simulink model "CCMSysServ"  (R2022b) (continued)
== system CCMSysServ == (continued)
  CarCfg_CarCfg250/1 -> CCMSysServ_Runnable_sys/247(CarCfg_CarCfg250_read)
  CarCfg_CarCfg253/1 -> CCMSysServ_Runnable_sys/248(CarCfg_CarCfg253_read)
  CarCfg_CarCfg254/1 -> CCMSysServ_Runnable_sys/249(CarCfg_CarCfg254_read)
  CarCfg_CarCfg255/1 -> CCMSysServ_Runnable_sys/250(CarCfg_CarCfg255_read)
  CarCfg_CarCfg256/1 -> CCMSysServ_Runnable_sys/251(CarCfg_CarCfg256_read)
  CarCfg_CarCfg257/1 -> CCMSysServ_Runnable_sys/252(CarCfg_CarCfg257_read)
  CarCfg_CarCfg258/1 -> CCMSysServ_Runnable_sys/253(CarCfg_CarCfg258_read)
  CarCfg_CarCfg259/1 -> CCMSysServ_Runnable_sys/254(CarCfg_CarCfg259_read)
  CarCfg_CarCfg262/1 -> CCMSysServ_Runnable_sys/255(CarCfg_CarCfg262_read)
  CarCfg_CarCfg263/1 -> CCMSysServ_Runnable_sys/256(CarCfg_CarCfg263_read)
  CarCfg_CarCfg267/1 -> CCMSysServ_Runnable_sys/257(CarCfg_CarCfg267_read)
  CarCfg_CarCfg268/1 -> CCMSysServ_Runnable_sys/258(CarCfg_CarCfg268_read)
  CarCfg_CarCfg270/1 -> CCMSysServ_Runnable_sys/259(CarCfg_CarCfg270_read)
  CarCfg_CarCfg271/1 -> CCMSysServ_Runnable_sys/260(CarCfg_CarCfg271_read)
  CarCfg_CarCfg273/1 -> CCMSysServ_Runnable_sys/261(CarCfg_CarCfg273_read)
  CarCfg_CarCfg274/1 -> CCMSysServ_Runnable_sys/262(CarCfg_CarCfg274_read)
  CarCfg_CarCfg275/1 -> CCMSysServ_Runnable_sys/263(CarCfg_CarCfg275_read)
  CarCfg_CarCfg28/1 -> CCMSysServ_Runnable_sys/264(CarCfg_CarCfg28_read)
  CarCfg_CarCfg289/1 -> CCMSysServ_Runnable_sys/265(CarCfg_CarCfg289_read)
  CarCfg_CarCfg290/1 -> CCMSysServ_Runnable_sys/266(CarCfg_CarCfg290_read)
  CarCfg_CarCfg3/1 -> CCMSysServ_Runnable_sys/267(CarCfg_CarCfg3_read)
  CarCfg_CarCfg30/1 -> CCMSysServ_Runnable_sys/268(CarCfg_CarCfg30_read)
  CarCfg_CarCfg301/1 -> CCMSysServ_Runnable_sys/269(CarCfg_CarCfg301_read)
  CarCfg_CarCfg302/1 -> CCMSysServ_Runnable_sys/270(CarCfg_CarCfg302_read)
  CarCfg_CarCfg303/1 -> CCMSysServ_Runnable_sys/271(CarCfg_CarCfg303_read)
  CarCfg_CarCfg304/1 -> CCMSysServ_Runnable_sys/272(CarCfg_CarCfg304_read)
  CarCfg_CarCfg305/1 -> CCMSysServ_Runnable_sys/273(CarCfg_CarCfg305_read)
  CarCfg_CarCfg307/1 -> CCMSysServ_Runnable_sys/274(CarCfg_CarCfg307_read)
  CarCfg_CarCfg308/1 -> CCMSysServ_Runnable_sys/275(CarCfg_CarCfg308_read)
  CarCfg_CarCfg309/1 -> CCMSysServ_Runnable_sys/276(CarCfg_CarCfg309_read)
  CarCfg_CarCfg310/1 -> CCMSysServ_Runnable_sys/277(CarCfg_CarCfg310_read)
  CarCfg_CarCfg311/1 -> CCMSysServ_Runnable_sys/278(CarCfg_CarCfg311_read)
  CarCfg_CarCfg312/1 -> CCMSysServ_Runnable_sys/279(CarCfg_CarCfg312_read)
  CarCfg_CarCfg313/1 -> CCMSysServ_Runnable_sys/280(CarCfg_CarCfg313_read)
  CarCfg_CarCfg314/1 -> CCMSysServ_Runnable_sys/281(CarCfg_CarCfg314_read)
  CarCfg_CarCfg315/1 -> CCMSysServ_Runnable_sys/282(CarCfg_CarCfg315_read)
  CarCfg_CarCfg316/1 -> CCMSysServ_Runnable_sys/283(CarCfg_CarCfg316_read)
  CarCfg_CarCfg317/1 -> CCMSysServ_Runnable_sys/284(CarCfg_CarCfg317_read)
  CarCfg_CarCfg318/1 -> CCMSysServ_Runnable_sys/285(CarCfg_CarCfg318_read)
  CarCfg_CarCfg319/1 -> CCMSysServ_Runnable_sys/286(CarCfg_CarCfg319_read)
  CarCfg_CarCfg32/1 -> CCMSysServ_Runnable_sys/287(CarCfg_CarCfg32_read)
  CarCfg_CarCfg321/1 -> CCMSysServ_Runnable_sys/288(CarCfg_CarCfg321_read)
  CarCfg_CarCfg322/1 -> CCMSysServ_Runnable_sys/289(CarCfg_CarCfg322_read)
  CarCfg_CarCfg323/1 -> CCMSysServ_Runnable_sys/290(CarCfg_CarCfg323_read)
  CarCfg_CarCfg324/1 -> CCMSysServ_Runnable_sys/291(CarCfg_CarCfg324_read)
  CarCfg_CarCfg325/1 -> CCMSysServ_Runnable_sys/292(CarCfg_CarCfg325_read)
  CarCfg_CarCfg33/1 -> CCMSysServ_Runnable_sys/293(CarCfg_CarCfg33_read)
  CarCfg_CarCfg335/1 -> CCMSysServ_Runnable_sys/294(CarCfg_CarCfg335_read)
  CarCfg_CarCfg336/1 -> CCMSysServ_Runnable_sys/295(CarCfg_CarCfg336_read)
  CarCfg_CarCfg337/1 -> CCMSysServ_Runnable_sys/296(CarCfg_CarCfg337_read)
  CarCfg_CarCfg338/1 -> CCMSysServ_Runnable_sys/297(CarCfg_CarCfg338_read)
  CarCfg_CarCfg339/1 -> CCMSysServ_Runnable_sys/298(CarCfg_CarCfg339_read)
  CarCfg_CarCfg34/1 -> CCMSysServ_Runnable_sys/299(CarCfg_CarCfg34_read)
  CarCfg_CarCfg340/1 -> CCMSysServ_Runnable_sys/300(CarCfg_CarCfg340_read)
  CarCfg_CarCfg341/1 -> CCMSysServ_Runnable_sys/301(CarCfg_CarCfg341_read)
  CarCfg_CarCfg342/1 -> CCMSysServ_Runnable_sys/302(CarCfg_CarCfg342_read)
  CarCfg_CarCfg343/1 -> CCMSysServ_Runnable_sys/303(CarCfg_CarCfg343_read)
  CarCfg_CarCfg344/1 -> CCMSysServ_Runnable_sys/304(CarCfg_CarCfg344_read)
  CarCfg_CarCfg345/1 -> CCMSysServ_Runnable_sys/305(CarCfg_CarCfg345_read)
  CarCfg_CarCfg346/1 -> CCMSysServ_Runnable_sys/306(CarCfg_CarCfg346_read)
  CarCfg_CarCfg347/1 -> CCMSysServ_Runnable_sys/307(CarCfg_CarCfg347_read)
  CarCfg_CarCfg348/1 -> CCMSysServ_Runnable_sys/308(CarCfg_CarCfg348_read)
  CarCfg_CarCfg349/1 -> CCMSysServ_Runnable_sys/309(CarCfg_CarCfg349_read)
  CarCfg_CarCfg35/1 -> CCMSysServ_Runnable_sys/310(CarCfg_CarCfg35_read)
  CarCfg_CarCfg350/1 -> CCMSysServ_Runnable_sys/311(CarCfg_CarCfg350_read)
  CarCfg_CarCfg351/1 -> CCMSysServ_Runnable_sys/312(CarCfg_CarCfg351_read)
  CarCfg_CarCfg352/1 -> CCMSysServ_Runnable_sys/313(CarCfg_CarCfg352_read)
  CarCfg_CarCfg353/1 -> CCMSysServ_Runnable_sys/314(CarCfg_CarCfg353_read)
  CarCfg_CarCfg354/1 -> CCMSysServ_Runnable_sys/315(CarCfg_CarCfg354_read)
  CarCfg_CarCfg355/1 -> CCMSysServ_Runnable_sys/316(CarCfg_CarCfg355_read)
  CarCfg_CarCfg356/1 -> CCMSysServ_Runnable_sys/317(CarCfg_CarCfg356_read)
  CarCfg_CarCfg357/1 -> CCMSysServ_Runnable_sys/318(CarCfg_CarCfg357_read)
  CarCfg_CarCfg358/1 -> CCMSysServ_Runnable_sys/319(CarCfg_CarCfg358_read)
  CarCfg_CarCfg359/1 -> CCMSysServ_Runnable_sys/320(CarCfg_CarCfg359_read)
  CarCfg_CarCfg36/1 -> CCMSysServ_Runnable_sys/321(CarCfg_CarCfg36_read)
  CarCfg_CarCfg360/1 -> CCMSysServ_Runnable_sys/322(CarCfg_CarCfg360_read)
  CarCfg_CarCfg361/1 -> CCMSysServ_Runnable_sys/323(CarCfg_CarCfg361_read)
  CarCfg_CarCfg362/1 -> CCMSysServ_Runnable_sys/324(CarCfg_CarCfg362_read)
  CarCfg_CarCfg363/1 -> CCMSysServ_Runnable_sys/325(CarCfg_CarCfg363_read)
  CarCfg_CarCfg364/1 -> CCMSysServ_Runnable_sys/326(CarCfg_CarCfg364_read)
  CarCfg_CarCfg365/1 -> CCMSysServ_Runnable_sys/327(CarCfg_CarCfg365_read)
  CarCfg_CarCfg366/1 -> CCMSysServ_Runnable_sys/328(CarCfg_CarCfg366_read)
  CarCfg_CarCfg367/1 -> CCMSysServ_Runnable_sys/329(CarCfg_CarCfg367_read)
  CarCfg_CarCfg368/1 -> CCMSysServ_Runnable_sys/330(CarCfg_CarCfg368_read)
  CarCfg_CarCfg369/1 -> CCMSysServ_Runnable_sys/331(CarCfg_CarCfg369_read)
  CarCfg_CarCfg37/1 -> CCMSysServ_Runnable_sys/332(CarCfg_CarCfg37_read)
  CarCfg_CarCfg371/1 -> CCMSysServ_Runnable_sys/333(CarCfg_CarCfg371_read)
  CarCfg_CarCfg372/1 -> CCMSysServ_Runnable_sys/334(CarCfg_CarCfg372_read)
  CarCfg_CarCfg373/1 -> CCMSysServ_Runnable_sys/335(CarCfg_CarCfg373_read)
  CarCfg_CarCfg374/1 -> CCMSysServ_Runnable_sys/336(CarCfg_CarCfg374_read)
  CarCfg_CarCfg376/1 -> CCMSysServ_Runnable_sys/337(CarCfg_CarCfg376_read)
  CarCfg_CarCfg38/1 -> CCMSysServ_Runnable_sys/338(CarCfg_CarCfg38_read)
  CarCfg_CarCfg39/1 -> CCMSysServ_Runnable_sys/339(CarCfg_CarCfg39_read)
  CarCfg_CarCfg4/1 -> CCMSysServ_Runnable_sys/340(CarCfg_CarCfg4_read)
  CarCfg_CarCfg401/1 -> CCMSysServ_Runnable_sys/341(CarCfg_CarCfg401_read)
  CarCfg_CarCfg407/1 -> CCMSysServ_Runnable_sys/342(CarCfg_CarCfg407_read)
  CarCfg_CarCfg408/1 -> CCMSysServ_Runnable_sys/343(CarCfg_CarCfg408_read)
  CarCfg_CarCfg409/1 -> CCMSysServ_Runnable_sys/344(CarCfg_CarCfg409_read)
  CarCfg_CarCfg41/1 -> CCMSysServ_Runnable_sys/345(CarCfg_CarCfg41_read)
  CarCfg_CarCfg410/1 -> CCMSysServ_Runnable_sys/346(CarCfg_CarCfg410_read)
  CarCfg_CarCfg413/1 -> CCMSysServ_Runnable_sys/347(CarCfg_CarCfg413_read)
  CarCfg_CarCfg414/1 -> CCMSysServ_Runnable_sys/348(CarCfg_CarCfg414_read)
  CarCfg_CarCfg415/1 -> CCMSysServ_Runnable_sys/349(CarCfg_CarCfg415_read)
  CarCfg_CarCfg42/1 -> CCMSysServ_Runnable_sys/350(CarCfg_CarCfg42_read)
  CarCfg_CarCfg43/1 -> CCMSysServ_Runnable_sys/351(CarCfg_CarCfg43_read)
  CarCfg_CarCfg44/1 -> CCMSysServ_Runnable_sys/352(CarCfg_CarCfg44_read)
  CarCfg_CarCfg441/1 -> CCMSysServ_Runnable_sys/353(CarCfg_CarCfg441_read)
  CarCfg_CarCfg449/1 -> CCMSysServ_Runnable_sys/354(CarCfg_CarCfg449_read)
  CarCfg_CarCfg45/1 -> CCMSysServ_Runnable_sys/355(CarCfg_CarCfg45_read)
  CarCfg_CarCfg450/1 -> CCMSysServ_Runnable_sys/356(CarCfg_CarCfg450_read)
  CarCfg_CarCfg451/1 -> CCMSysServ_Runnable_sys/357(CarCfg_CarCfg451_read)
  CarCfg_CarCfg452/1 -> CCMSysServ_Runnable_sys/358(CarCfg_CarCfg452_read)
  CarCfg_CarCfg453/1 -> CCMSysServ_Runnable_sys/359(CarCfg_CarCfg453_read)
  CarCfg_CarCfg454/1 -> CCMSysServ_Runnable_sys/360(CarCfg_CarCfg454_read)
  CarCfg_CarCfg455/1 -> CCMSysServ_Runnable_sys/361(CarCfg_CarCfg455_read)
  CarCfg_CarCfg456/1 -> CCMSysServ_Runnable_sys/362(CarCfg_CarCfg456_read)
  CarCfg_CarCfg457/1 -> CCMSysServ_Runnable_sys/363(CarCfg_CarCfg457_read)
  CarCfg_CarCfg458/1 -> CCMSysServ_Runnable_sys/364(CarCfg_CarCfg458_read)
  CarCfg_CarCfg459/1 -> CCMSysServ_Runnable_sys/365(CarCfg_CarCfg459_read)
  CarCfg_CarCfg46/1 -> CCMSysServ_Runnable_sys/366(CarCfg_CarCfg46_read)
  CarCfg_CarCfg460/1 -> CCMSysServ_Runnable_sys/367(CarCfg_CarCfg460_read)
  CarCfg_CarCfg461/1 -> CCMSysServ_Runnable_sys/368(CarCfg_CarCfg461_read)
  CarCfg_CarCfg462/1 -> CCMSysServ_Runnable_sys/369(CarCfg_CarCfg462_read)
  CarCfg_CarCfg463/1 -> CCMSysServ_Runnable_sys/370(CarCfg_CarCfg463_read)
  CarCfg_CarCfg464/1 -> CCMSysServ_Runnable_sys/371(CarCfg_CarCfg464_read)
  CarCfg_CarCfg465/1 -> CCMSysServ_Runnable_sys/372(CarCfg_CarCfg465_read)
  CarCfg_CarCfg466/1 -> CCMSysServ_Runnable_sys/373(CarCfg_CarCfg466_read)
  CarCfg_CarCfg467/1 -> CCMSysServ_Runnable_sys/374(CarCfg_CarCfg467_read)
  CarCfg_CarCfg468/1 -> CCMSysServ_Runnable_sys/375(CarCfg_CarCfg468_read)
  CarCfg_CarCfg469/1 -> CCMSysServ_Runnable_sys/376(CarCfg_CarCfg469_read)
  CarCfg_CarCfg47/1 -> CCMSysServ_Runnable_sys/377(CarCfg_CarCfg47_read)
  CarCfg_CarCfg470/1 -> CCMSysServ_Runnable_sys/378(CarCfg_CarCfg470_read)
  CarCfg_CarCfg471/1 -> CCMSysServ_Runnable_sys/379(CarCfg_CarCfg471_read)
  CarCfg_CarCfg472/1 -> CCMSysServ_Runnable_sys/380(CarCfg_CarCfg472_read)
  CarCfg_CarCfg473/1 -> CCMSysServ_Runnable_sys/381(CarCfg_CarCfg473_read)
  CarCfg_CarCfg474/1 -> CCMSysServ_Runnable_sys/382(CarCfg_CarCfg474_read)
  CarCfg_CarCfg475/1 -> CCMSysServ_Runnable_sys/383(CarCfg_CarCfg475_read)
  CarCfg_CarCfg476/1 -> CCMSysServ_Runnable_sys/384(CarCfg_CarCfg476_read)
  CarCfg_CarCfg477/1 -> CCMSysServ_Runnable_sys/385(CarCfg_CarCfg477_read)
  CarCfg_CarCfg478/1 -> CCMSysServ_Runnable_sys/386(CarCfg_CarCfg478_read)
  CarCfg_CarCfg479/1 -> CCMSysServ_Runnable_sys/387(CarCfg_CarCfg479_read)
  CarCfg_CarCfg48/1 -> CCMSysServ_Runnable_sys/388(CarCfg_CarCfg48_read)
  CarCfg_CarCfg480/1 -> CCMSysServ_Runnable_sys/389(CarCfg_CarCfg480_read)
  CarCfg_CarCfg481/1 -> CCMSysServ_Runnable_sys/390(CarCfg_CarCfg481_read)
  CarCfg_CarCfg482/1 -> CCMSysServ_Runnable_sys/391(CarCfg_CarCfg482_read)
  CarCfg_CarCfg483/1 -> CCMSysServ_Runnable_sys/392(CarCfg_CarCfg483_read)
  CarCfg_CarCfg484/1 -> CCMSysServ_Runnable_sys/393(CarCfg_CarCfg484_read)
  CarCfg_CarCfg485/1 -> CCMSysServ_Runnable_sys/394(CarCfg_CarCfg485_read)
  CarCfg_CarCfg486/1 -> CCMSysServ_Runnable_sys/395(CarCfg_CarCfg486_read)
  CarCfg_CarCfg487/1 -> CCMSysServ_Runnable_sys/396(CarCfg_CarCfg487_read)
  CarCfg_CarCfg488/1 -> CCMSysServ_Runnable_sys/397(CarCfg_CarCfg488_read)
  CarCfg_CarCfg489/1 -> CCMSysServ_Runnable_sys/398(CarCfg_CarCfg489_read)
  CarCfg_CarCfg49/1 -> CCMSysServ_Runnable_sys/399(CarCfg_CarCfg49_read)
  CarCfg_CarCfg490/1 -> CCMSysServ_Runnable_sys/400(CarCfg_CarCfg490_read)
  CarCfg_CarCfg491/1 -> CCMSysServ_Runnable_sys/401(CarCfg_CarCfg491_read)
  CarCfg_CarCfg493/1 -> CCMSysServ_Runnable_sys/402(CarCfg_CarCfg493_read)
  CarCfg_CarCfg494/1 -> CCMSysServ_Runnable_sys/403(CarCfg_CarCfg494_read)
  CarCfg_CarCfg495/1 -> CCMSysServ_Runnable_sys/404(CarCfg_CarCfg495_read)
  CarCfg_CarCfg496/1 -> CCMSysServ_Runnable_sys/405(CarCfg_CarCfg496_read)
  CarCfg_CarCfg497/1 -> CCMSysServ_Runnable_sys/406(CarCfg_CarCfg497_read)
  CarCfg_CarCfg498/1 -> CCMSysServ_Runnable_sys/407(CarCfg_CarCfg498_read)
  CarCfg_CarCfg499/1 -> CCMSysServ_Runnable_sys/408(CarCfg_CarCfg499_read)
  CarCfg_CarCfg5/1 -> CCMSysServ_Runnable_sys/409(CarCfg_CarCfg5_read)
  CarCfg_CarCfg50/1 -> CCMSysServ_Runnable_sys/410(CarCfg_CarCfg50_read)
  CarCfg_CarCfg500/1 -> CCMSysServ_Runnable_sys/411(CarCfg_CarCfg500_read)
  CarCfg_CarCfg501/1 -> CCMSysServ_Runnable_sys/412(CarCfg_CarCfg501_read)
  CarCfg_CarCfg502/1 -> CCMSysServ_Runnable_sys/413(CarCfg_CarCfg502_read)
  CarCfg_CarCfg503/1 -> CCMSysServ_Runnable_sys/414(CarCfg_CarCfg503_read)
  CarCfg_CarCfg504/1 -> CCMSysServ_Runnable_sys/415(CarCfg_CarCfg504_read)
  CarCfg_CarCfg505/1 -> CCMSysServ_Runnable_sys/416(CarCfg_CarCfg505_read)
  CarCfg_CarCfg506/1 -> CCMSysServ_Runnable_sys/417(CarCfg_CarCfg506_read)
  CarCfg_CarCfg507/1 -> CCMSysServ_Runnable_sys/418(CarCfg_CarCfg507_read)
  CarCfg_CarCfg508/1 -> CCMSysServ_Runnable_sys/419(CarCfg_CarCfg508_read)
  CarCfg_CarCfg509/1 -> CCMSysServ_Runnable_sys/420(CarCfg_CarCfg509_read)
  CarCfg_CarCfg51/1 -> CCMSysServ_Runnable_sys/421(CarCfg_CarCfg51_read)
  CarCfg_CarCfg510/1 -> CCMSysServ_Runnable_sys/422(CarCfg_CarCfg510_read)
  CarCfg_CarCfg511/1 -> CCMSysServ_Runnable_sys/423(CarCfg_CarCfg511_read)
  CarCfg_CarCfg512/1 -> CCMSysServ_Runnable_sys/424(CarCfg_CarCfg512_read)
  CarCfg_CarCfg513/1 -> CCMSysServ_Runnable_sys/425(CarCfg_CarCfg513_read)
  CarCfg_CarCfg514/1 -> CCMSysServ_Runnable_sys/426(CarCfg_CarCfg514_read)
  CarCfg_CarCfg515/1 -> CCMSysServ_Runnable_sys/427(CarCfg_CarCfg515_read)
  CarCfg_CarCfg516/1 -> CCMSysServ_Runnable_sys/428(CarCfg_CarCfg516_read)
  CarCfg_CarCfg517/1 -> CCMSysServ_Runnable_sys/429(CarCfg_CarCfg517_read)
  CarCfg_CarCfg518/1 -> CCMSysServ_Runnable_sys/430(CarCfg_CarCfg518_read)
  CarCfg_CarCfg519/1 -> CCMSysServ_Runnable_sys/431(CarCfg_CarCfg519_read)
  CarCfg_CarCfg52/1 -> CCMSysServ_Runnable_sys/432(CarCfg_CarCfg52_read)
  CarCfg_CarCfg520/1 -> CCMSysServ_Runnable_sys/433(CarCfg_CarCfg520_read)
  CarCfg_CarCfg521/1 -> CCMSysServ_Runnable_sys/434(CarCfg_CarCfg521_read)
  CarCfg_CarCfg522/1 -> CCMSysServ_Runnable_sys/435(CarCfg_CarCfg522_read)
  CarCfg_CarCfg523/1 -> CCMSysServ_Runnable_sys/436(CarCfg_CarCfg523_read)
  CarCfg_CarCfg524/1 -> CCMSysServ_Runnable_sys/437(CarCfg_CarCfg524_read)
  CarCfg_CarCfg525/1 -> CCMSysServ_Runnable_sys/438(CarCfg_CarCfg525_read)
  CarCfg_CarCfg526/1 -> CCMSysServ_Runnable_sys/439(CarCfg_CarCfg526_read)
  CarCfg_CarCfg527/1 -> CCMSysServ_Runnable_sys/440(CarCfg_CarCfg527_read)
  CarCfg_CarCfg528/1 -> CCMSysServ_Runnable_sys/441(CarCfg_CarCfg528_read)
  CarCfg_CarCfg529/1 -> CCMSysServ_Runnable_sys/442(CarCfg_CarCfg529_read)
  CarCfg_CarCfg53/1 -> CCMSysServ_Runnable_sys/443(CarCfg_CarCfg53_read)
  CarCfg_CarCfg531/1 -> CCMSysServ_Runnable_sys/444(CarCfg_CarCfg531_read)
  CarCfg_CarCfg532/1 -> CCMSysServ_Runnable_sys/445(CarCfg_CarCfg532_read)
  CarCfg_CarCfg533/1 -> CCMSysServ_Runnable_sys/446(CarCfg_CarCfg533_read)
  CarCfg_CarCfg534/1 -> CCMSysServ_Runnable_sys/447(CarCfg_CarCfg534_read)
  CarCfg_CarCfg535/1 -> CCMSysServ_Runnable_sys/448(CarCfg_CarCfg535_read)
  CarCfg_CarCfg536/1 -> CCMSysServ_Runnable_sys/449(CarCfg_CarCfg536_read)
  CarCfg_CarCfg54/1 -> CCMSysServ_Runnable_sys/450(CarCfg_CarCfg54_read)
  CarCfg_CarCfg540/1 -> CCMSysServ_Runnable_sys/451(CarCfg_CarCfg540_read)
  CarCfg_CarCfg541/1 -> CCMSysServ_Runnable_sys/452(CarCfg_CarCfg541_read)
  CarCfg_CarCfg543/1 -> CCMSysServ_Runnable_sys/453(CarCfg_CarCfg543_read)
  CarCfg_CarCfg544/1 -> CCMSysServ_Runnable_sys/454(CarCfg_CarCfg544_read)
  CarCfg_CarCfg545/1 -> CCMSysServ_Runnable_sys/455(CarCfg_CarCfg545_read)
  CarCfg_CarCfg546/1 -> CCMSysServ_Runnable_sys/456(CarCfg_CarCfg546_read)
  CarCfg_CarCfg547/1 -> CCMSysServ_Runnable_sys/457(CarCfg_CarCfg547_read)
  CarCfg_CarCfg55/1 -> CCMSysServ_Runnable_sys/458(CarCfg_CarCfg55_read)
  CarCfg_CarCfg550/1 -> CCMSysServ_Runnable_sys/459(CarCfg_CarCfg550_read)
  CarCfg_CarCfg551/1 -> CCMSysServ_Runnable_sys/460(CarCfg_CarCfg551_read)
  CarCfg_CarCfg56/1 -> CCMSysServ_Runnable_sys/461(CarCfg_CarCfg56_read)
  CarCfg_CarCfg561/1 -> CCMSysServ_Runnable_sys/462(CarCfg_CarCfg561_read)
  CarCfg_CarCfg564/1 -> CCMSysServ_Runnable_sys/463(CarCfg_CarCfg564_read)
  CarCfg_CarCfg565/1 -> CCMSysServ_Runnable_sys/464(CarCfg_CarCfg565_read)
  CarCfg_CarCfg566/1 -> CCMSysServ_Runnable_sys/465(CarCfg_CarCfg566_read)
  CarCfg_CarCfg567/1 -> CCMSysServ_Runnable_sys/466(CarCfg_CarCfg567_read)
  CarCfg_CarCfg568/1 -> CCMSysServ_Runnable_sys/467(CarCfg_CarCfg568_read)
  CarCfg_CarCfg569/1 -> CCMSysServ_Runnable_sys/468(CarCfg_CarCfg569_read)
  CarCfg_CarCfg57/1 -> CCMSysServ_Runnable_sys/469(CarCfg_CarCfg57_read)
  CarCfg_CarCfg578/1 -> CCMSysServ_Runnable_sys/470(CarCfg_CarCfg578_read)
  CarCfg_CarCfg579/1 -> CCMSysServ_Runnable_sys/471(CarCfg_CarCfg579_read)
  CarCfg_CarCfg58/1 -> CCMSysServ_Runnable_sys/472(CarCfg_CarCfg58_read)
  CarCfg_CarCfg580/1 -> CCMSysServ_Runnable_sys/473(CarCfg_CarCfg580_read)
  CarCfg_CarCfg59/1 -> CCMSysServ_Runnable_sys/474(CarCfg_CarCfg59_read)
  CarCfg_CarCfg590/1 -> CCMSysServ_Runnable_sys/475(CarCfg_CarCfg590_read)
  CarCfg_CarCfg591/1 -> CCMSysServ_Runnable_sys/476(CarCfg_CarCfg591_read)
  CarCfg_CarCfg593/1 -> CCMSysServ_Runnable_sys/477(CarCfg_CarCfg593_read)
  CarCfg_CarCfg595/1 -> CCMSysServ_Runnable_sys/478(CarCfg_CarCfg595_read)
  CarCfg_CarCfg599/1 -> CCMSysServ_Runnable_sys/479(CarCfg_CarCfg599_read)
  CarCfg_CarCfg6/1 -> CCMSysServ_Runnable_sys/480(CarCfg_CarCfg6_read)
  CarCfg_CarCfg60/1 -> CCMSysServ_Runnable_sys/481(CarCfg_CarCfg60_read)
  CarCfg_CarCfg604/1 -> CCMSysServ_Runnable_sys/482(CarCfg_CarCfg604_read)
  CarCfg_CarCfg609/1 -> CCMSysServ_Runnable_sys/483(CarCfg_CarCfg609_read)
  CarCfg_CarCfg61/1 -> CCMSysServ_Runnable_sys/484(CarCfg_CarCfg61_read)
  CarCfg_CarCfg611/1 -> CCMSysServ_Runnable_sys/485(CarCfg_CarCfg611_read)
  CarCfg_CarCfg613/1 -> CCMSysServ_Runnable_sys/486(CarCfg_CarCfg613_read)
  CarCfg_CarCfg615/1 -> CCMSysServ_Runnable_sys/487(CarCfg_CarCfg615_read)
  CarCfg_CarCfg616/1 -> CCMSysServ_Runnable_sys/488(CarCfg_CarCfg616_read)
  CarCfg_CarCfg617/1 -> CCMSysServ_Runnable_sys/489(CarCfg_CarCfg617_read)
  CarCfg_CarCfg618/1 -> CCMSysServ_Runnable_sys/490(CarCfg_CarCfg618_read)
  CarCfg_CarCfg62/1 -> CCMSysServ_Runnable_sys/491(CarCfg_CarCfg62_read)
  CarCfg_CarCfg622/1 -> CCMSysServ_Runnable_sys/492(CarCfg_CarCfg622_read)
  CarCfg_CarCfg624/1 -> CCMSysServ_Runnable_sys/493(CarCfg_CarCfg624_read)
  CarCfg_CarCfg626/1 -> CCMSysServ_Runnable_sys/494(CarCfg_CarCfg626_read)
  CarCfg_CarCfg627/1 -> CCMSysServ_Runnable_sys/495(CarCfg_CarCfg627_read)
  CarCfg_CarCfg628/1 -> CCMSysServ_Runnable_sys/496(CarCfg_CarCfg628_read)
  CarCfg_CarCfg629/1 -> CCMSysServ_Runnable_sys/497(CarCfg_CarCfg629_read)
  CarCfg_CarCfg63/1 -> CCMSysServ_Runnable_sys/498(CarCfg_CarCfg63_read)
  CarCfg_CarCfg630/1 -> CCMSysServ_Runnable_sys/499(CarCfg_CarCfg630_read)
  CarCfg_CarCfg634/1 -> CCMSysServ_Runnable_sys/500(CarCfg_CarCfg634_read)
  CarCfg_CarCfg635/1 -> CCMSysServ_Runnable_sys/501(CarCfg_CarCfg635_read)
  CarCfg_CarCfg639/1 -> CCMSysServ_Runnable_sys/502(CarCfg_CarCfg639_read)
  CarCfg_CarCfg64/1 -> CCMSysServ_Runnable_sys/503(CarCfg_CarCfg64_read)
  CarCfg_CarCfg640/1 -> CCMSysServ_Runnable_sys/504(CarCfg_CarCfg640_read)
  CarCfg_CarCfg642/1 -> CCMSysServ_Runnable_sys/505(CarCfg_CarCfg642_read)
  CarCfg_CarCfg644/1 -> CCMSysServ_Runnable_sys/506(CarCfg_CarCfg644_read)
  CarCfg_CarCfg645/1 -> CCMSysServ_Runnable_sys/507(CarCfg_CarCfg645_read)
  CarCfg_CarCfg646/1 -> CCMSysServ_Runnable_sys/508(CarCfg_CarCfg646_read)
  CarCfg_CarCfg647/1 -> CCMSysServ_Runnable_sys/509(CarCfg_CarCfg647_read)
  CarCfg_CarCfg648/1 -> CCMSysServ_Runnable_sys/510(CarCfg_CarCfg648_read)
  CarCfg_CarCfg65/1 -> CCMSysServ_Runnable_sys/511(CarCfg_CarCfg65_read)
  CarCfg_CarCfg650/1 -> CCMSysServ_Runnable_sys/512(CarCfg_CarCfg650_read)
  CarCfg_CarCfg651/1 -> CCMSysServ_Runnable_sys/513(CarCfg_CarCfg651_read)
  CarCfg_CarCfg652/1 -> CCMSysServ_Runnable_sys/514(CarCfg_CarCfg652_read)
  CarCfg_CarCfg659/1 -> CCMSysServ_Runnable_sys/515(CarCfg_CarCfg659_read)
  CarCfg_CarCfg66/1 -> CCMSysServ_Runnable_sys/516(CarCfg_CarCfg66_read)
  CarCfg_CarCfg660/1 -> CCMSysServ_Runnable_sys/517(CarCfg_CarCfg660_read)
  CarCfg_CarCfg665/1 -> CCMSysServ_Runnable_sys/518(CarCfg_CarCfg665_read)
  CarCfg_CarCfg67/1 -> CCMSysServ_Runnable_sys/519(CarCfg_CarCfg67_read)
  CarCfg_CarCfg671/1 -> CCMSysServ_Runnable_sys/520(CarCfg_CarCfg671_read)
  CarCfg_CarCfg672/1 -> CCMSysServ_Runnable_sys/521(CarCfg_CarCfg672_read)
  CarCfg_CarCfg675/1 -> CCMSysServ_Runnable_sys/522(CarCfg_CarCfg675_read)
  CarCfg_CarCfg677/1 -> CCMSysServ_Runnable_sys/523(CarCfg_CarCfg677_read)
  CarCfg_CarCfg678/1 -> CCMSysServ_Runnable_sys/524(CarCfg_CarCfg678_read)
  CarCfg_CarCfg68/1 -> CCMSysServ_Runnable_sys/525(CarCfg_CarCfg68_read)
  CarCfg_CarCfg683/1 -> CCMSysServ_Runnable_sys/526(CarCfg_CarCfg683_read)
  CarCfg_CarCfg684/1 -> CCMSysServ_Runnable_sys/527(CarCfg_CarCfg684_read)
  CarCfg_CarCfg686/1 -> CCMSysServ_Runnable_sys/528(CarCfg_CarCfg686_read)
  CarCfg_CarCfg687/1 -> CCMSysServ_Runnable_sys/529(CarCfg_CarCfg687_read)
  CarCfg_CarCfg688/1 -> CCMSysServ_Runnable_sys/530(CarCfg_CarCfg688_read)
  CarCfg_CarCfg69/1 -> CCMSysServ_Runnable_sys/531(CarCfg_CarCfg69_read)
  CarCfg_CarCfg695/1 -> CCMSysServ_Runnable_sys/532(CarCfg_CarCfg695_read)
  CarCfg_CarCfg697/1 -> CCMSysServ_Runnable_sys/533(CarCfg_CarCfg697_read)
  CarCfg_CarCfg698/1 -> CCMSysServ_Runnable_sys/534(CarCfg_CarCfg698_read)
  CarCfg_CarCfg699/1 -> CCMSysServ_Runnable_sys/535(CarCfg_CarCfg699_read)
  CarCfg_CarCfg70/1 -> CCMSysServ_Runnable_sys/536(CarCfg_CarCfg70_read)
  CarCfg_CarCfg701/1 -> CCMSysServ_Runnable_sys/537(CarCfg_CarCfg701_read)
  CarCfg_CarCfg705/1 -> CCMSysServ_Runnable_sys/538(CarCfg_CarCfg705_read)
  CarCfg_CarCfg709/1 -> CCMSysServ_Runnable_sys/539(CarCfg_CarCfg709_read)
  CarCfg_CarCfg71/1 -> CCMSysServ_Runnable_sys/540(CarCfg_CarCfg71_read)
  CarCfg_CarCfg714/1 -> CCMSysServ_Runnable_sys/541(CarCfg_CarCfg714_read)
  CarCfg_CarCfg715/1 -> CCMSysServ_Runnable_sys/542(CarCfg_CarCfg715_read)
  CarCfg_CarCfg718/1 -> CCMSysServ_Runnable_sys/543(CarCfg_CarCfg718_read)
  CarCfg_CarCfg719/1 -> CCMSysServ_Runnable_sys/544(CarCfg_CarCfg719_read)
  CarCfg_CarCfg72/1 -> CCMSysServ_Runnable_sys/545(CarCfg_CarCfg72_read)
  CarCfg_CarCfg720/1 -> CCMSysServ_Runnable_sys/546(CarCfg_CarCfg720_read)
  CarCfg_CarCfg721/1 -> CCMSysServ_Runnable_sys/547(CarCfg_CarCfg721_read)
  CarCfg_CarCfg726/1 -> CCMSysServ_Runnable_sys/548(CarCfg_CarCfg726_read)
  CarCfg_CarCfg73/1 -> CCMSysServ_Runnable_sys/549(CarCfg_CarCfg73_read)
  CarCfg_CarCfg732/1 -> CCMSysServ_Runnable_sys/550(CarCfg_CarCfg732_read)
  CarCfg_CarCfg733/1 -> CCMSysServ_Runnable_sys/551(CarCfg_CarCfg733_read)
  CarCfg_CarCfg734/1 -> CCMSysServ_Runnable_sys/552(CarCfg_CarCfg734_read)
  CarCfg_CarCfg74/1 -> CCMSysServ_Runnable_sys/553(CarCfg_CarCfg74_read)
  CarCfg_CarCfg740/1 -> CCMSysServ_Runnable_sys/554(CarCfg_CarCfg740_read)
  CarCfg_CarCfg741/1 -> CCMSysServ_Runnable_sys/555(CarCfg_CarCfg741_read)
  CarCfg_CarCfg742/1 -> CCMSysServ_Runnable_sys/556(CarCfg_CarCfg742_read)
  CarCfg_CarCfg746/1 -> CCMSysServ_Runnable_sys/557(CarCfg_CarCfg746_read)
  CarCfg_CarCfg75/1 -> CCMSysServ_Runnable_sys/558(CarCfg_CarCfg75_read)
  CarCfg_CarCfg753/1 -> CCMSysServ_Runnable_sys/559(CarCfg_CarCfg753_read)
  CarCfg_CarCfg755/1 -> CCMSysServ_Runnable_sys/560(CarCfg_CarCfg755_read)
  CarCfg_CarCfg756/1 -> CCMSysServ_Runnable_sys/561(CarCfg_CarCfg756_read)
  CarCfg_CarCfg76/1 -> CCMSysServ_Runnable_sys/562(CarCfg_CarCfg76_read)
  CarCfg_CarCfg763/1 -> CCMSysServ_Runnable_sys/563(CarCfg_CarCfg763_read)
  CarCfg_CarCfg766/1 -> CCMSysServ_Runnable_sys/564(CarCfg_CarCfg766_read)
  CarCfg_CarCfg768/1 -> CCMSysServ_Runnable_sys/565(CarCfg_CarCfg768_read)
  CarCfg_CarCfg77/1 -> CCMSysServ_Runnable_sys/566(CarCfg_CarCfg77_read)
  CarCfg_CarCfg770/1 -> CCMSysServ_Runnable_sys/567(CarCfg_CarCfg770_read)
  CarCfg_CarCfg773/1 -> CCMSysServ_Runnable_sys/568(CarCfg_CarCfg773_read)
  CarCfg_CarCfg776/1 -> CCMSysServ_Runnable_sys/569(CarCfg_CarCfg776_read)
  CarCfg_CarCfg779/1 -> CCMSysServ_Runnable_sys/570(CarCfg_CarCfg779_read)
  CarCfg_CarCfg78/1 -> CCMSysServ_Runnable_sys/571(CarCfg_CarCfg78_read)
  CarCfg_CarCfg787/1 -> CCMSysServ_Runnable_sys/572(CarCfg_CarCfg787_read)
  CarCfg_CarCfg789/1 -> CCMSysServ_Runnable_sys/573(CarCfg_CarCfg789_read)
  CarCfg_CarCfg79/1 -> CCMSysServ_Runnable_sys/574(CarCfg_CarCfg79_read)
  CarCfg_CarCfg790/1 -> CCMSysServ_Runnable_sys/575(CarCfg_CarCfg790_read)
  CarCfg_CarCfg793/1 -> CCMSysServ_Runnable_sys/576(CarCfg_CarCfg793_read)
  CarCfg_CarCfg794/1 -> CCMSysServ_Runnable_sys/577(CarCfg_CarCfg794_read)
  CarCfg_CarCfg798/1 -> CCMSysServ_Runnable_sys/578(CarCfg_CarCfg798_read)
  CarCfg_CarCfg8/1 -> CCMSysServ_Runnable_sys/579(CarCfg_CarCfg8_read)
  CarCfg_CarCfg80/1 -> CCMSysServ_Runnable_sys/580(CarCfg_CarCfg80_read)
  CarCfg_CarCfg81/1 -> CCMSysServ_Runnable_sys/581(CarCfg_CarCfg81_read)
  CarCfg_CarCfg811/1 -> CCMSysServ_Runnable_sys/582(CarCfg_CarCfg811_read)
  CarCfg_CarCfg814/1 -> CCMSysServ_Runnable_sys/583(CarCfg_CarCfg814_read)
  CarCfg_CarCfg816/1 -> CCMSysServ_Runnable_sys/584(CarCfg_CarCfg816_read)
  CarCfg_CarCfg82/1 -> CCMSysServ_Runnable_sys/585(CarCfg_CarCfg82_read)
  CarCfg_CarCfg822/1 -> CCMSysServ_Runnable_sys/586(CarCfg_CarCfg822_read)
  CarCfg_CarCfg826/1 -> CCMSysServ_Runnable_sys/587(CarCfg_CarCfg826_read)
  CarCfg_CarCfg829/1 -> CCMSysServ_Runnable_sys/588(CarCfg_CarCfg829_read)
  CarCfg_CarCfg83/1 -> CCMSysServ_Runnable_sys/589(CarCfg_CarCfg83_read)
  CarCfg_CarCfg831/1 -> CCMSysServ_Runnable_sys/590(CarCfg_CarCfg831_read)
  CarCfg_CarCfg834/1 -> CCMSysServ_Runnable_sys/591(CarCfg_CarCfg834_read)
  CarCfg_CarCfg835/1 -> CCMSysServ_Runnable_sys/592(CarCfg_CarCfg835_read)
  CarCfg_CarCfg837/1 -> CCMSysServ_Runnable_sys/593(CarCfg_CarCfg837_read)
  CarCfg_CarCfg839/1 -> CCMSysServ_Runnable_sys/594(CarCfg_CarCfg839_read)
  CarCfg_CarCfg84/1 -> CCMSysServ_Runnable_sys/595(CarCfg_CarCfg84_read)
  CarCfg_CarCfg841/1 -> CCMSysServ_Runnable_sys/596(CarCfg_CarCfg841_read)
  CarCfg_CarCfg842/1 -> CCMSysServ_Runnable_sys/597(CarCfg_CarCfg842_read)
  CarCfg_CarCfg846/1 -> CCMSysServ_Runnable_sys/598(CarCfg_CarCfg846_read)
  CarCfg_CarCfg848/1 -> CCMSysServ_Runnable_sys/599(CarCfg_CarCfg848_read)
  CarCfg_CarCfg849/1 -> CCMSysServ_Runnable_sys/600(CarCfg_CarCfg849_read)
  CarCfg_CarCfg85/1 -> CCMSysServ_Runnable_sys/601(CarCfg_CarCfg85_read)
  CarCfg_CarCfg851/1 -> CCMSysServ_Runnable_sys/602(CarCfg_CarCfg851_read)
  CarCfg_CarCfg855/1 -> CCMSysServ_Runnable_sys/603(CarCfg_CarCfg855_read)
  CarCfg_CarCfg857/1 -> CCMSysServ_Runnable_sys/604(CarCfg_CarCfg857_read)
  CarCfg_CarCfg858/1 -> CCMSysServ_Runnable_sys/605(CarCfg_CarCfg858_read)
  CarCfg_CarCfg86/1 -> CCMSysServ_Runnable_sys/606(CarCfg_CarCfg86_read)
  CarCfg_CarCfg87/1 -> CCMSysServ_Runnable_sys/607(CarCfg_CarCfg87_read)
  CarCfg_CarCfg88/1 -> CCMSysServ_Runnable_sys/608(CarCfg_CarCfg88_read)
  CarCfg_CarCfg89/1 -> CCMSysServ_Runnable_sys/609(CarCfg_CarCfg89_read)
  CarCfg_CarCfg9/1 -> CCMSysServ_Runnable_sys/610(CarCfg_CarCfg9_read)
  CarCfg_CarCfg90/1 -> CCMSysServ_Runnable_sys/611(CarCfg_CarCfg90_read)
  CarCfg_CarCfg91/1 -> CCMSysServ_Runnable_sys/612(CarCfg_CarCfg91_read)
  CarCfg_CarCfg92/1 -> CCMSysServ_Runnable_sys/613(CarCfg_CarCfg92_read)
  CarCfg_CarCfg93/1 -> CCMSysServ_Runnable_sys/614(CarCfg_CarCfg93_read)
  CarCfg_CarCfg94/1 -> CCMSysServ_Runnable_sys/615(CarCfg_CarCfg94_read)
  CarCfg_CarCfg95/1 -> CCMSysServ_Runnable_sys/616(CarCfg_CarCfg95_read)
  CarCfg_CarCfg96/1 -> CCMSysServ_Runnable_sys/617(CarCfg_CarCfg96_read)
  CarCfg_CarCfg97/1 -> CCMSysServ_Runnable_sys/618(CarCfg_CarCfg97_read)
  CarCfg_CarCfg98/1 -> CCMSysServ_Runnable_sys/619(CarCfg_CarCfg98_read)
  CarCfg_CarCfg99/1 -> CCMSysServ_Runnable_sys/620(CarCfg_CarCfg99_read)
  CCMSysServ_Runnable_sys/1(CarCfgForClimaCtrl_CarCfgFor3RDROWSEATHEAT_write) -> CarCfgForClimaCtrl_CarCfgFor3RDROWSEATHEAT/1
  CCMSysServ_Runnable_sys/2(CarCfgForClimaCtrl_CarCfgFor3RDROWSEATVENTILATED_write) -> CarCfgForClimaCtrl_CarCfgFor3RDROWSEATVENTILATED/1
  CCMSysServ_Runnable_sys/3(CarCfgForClimaCtrl_CarCfgForBRAND_write) -> CarCfgForClimaCtrl_CarCfgForBRAND/1
  CCMSysServ_Runnable_sys/4(CarCfgForClimaCtrl_CarCfgForCabinOxgenSupply_write) -> CarCfgForClimaCtrl_CarCfgForCabinOxgenSupply/1
  CCMSysServ_Runnable_sys/5(CarCfgForClimaCtrl_CarCfgForElectricLiftingModule_write) -> CarCfgForClimaCtrl_CarCfgForElectricLiftingModule/1
  CCMSysServ_Runnable_sys/6(CarCfgForClimaCtrl_CarCfgForExtVentnClimaCtrl_write) -> CarCfgForClimaCtrl_CarCfgForExtVentnClimaCtrl/1
  CCMSysServ_Runnable_sys/7(CarCfgForClimaCtrl_CarCfgForTEMPEREDSTORAGEBOX_write) -> CarCfgForClimaCtrl_CarCfgForTEMPEREDSTORAGEBOX/1
  CCMSysServ_Runnable_sys/8(CarCfgForClimaCtrl_CarCfgForFloatingDriving_write) -> CarCfgForClimaCtrl_CarCfgForFloatingDriving/1
  CCMSysServ_Runnable_sys/9(CarCfgForClimaCtrl_CarCfgForInteriorViewCamera_write) -> CarCfgForClimaCtrl_CarCfgForInteriorViewCamera/1
  CCMSysServ_Runnable_sys/10(CarCfgForClimaCtrl_CarCfgForLifeDetection_write) -> CarCfgForClimaCtrl_CarCfgForLifeDetection/1
  CCMSysServ_Runnable_sys/11(CarCfgForClimaCtrl_CarCfgForMARKET_write) -> CarCfgForClimaCtrl_CarCfgForMARKET/1
  CCMSysServ_Runnable_sys/12(CarCfgForClimaCtrl_CarCfgForPowerSeats_write) -> CarCfgForClimaCtrl_CarCfgForPowerSeats/1
  CCMSysServ_Runnable_sys/13(CarCfgForClimaCtrl_CarCfgForPTCInFrontHVAC_write) -> CarCfgForClimaCtrl_CarCfgForPTCInFrontHVAC/1
  CCMSysServ_Runnable_sys/14(CarCfgForClimaCtrl_CarCfgForRFKEY_write) -> CarCfgForClimaCtrl_CarCfgForRFKEY/1
  CCMSysServ_Runnable_sys/15(CarCfgForClimaCtrl_CarCfgForVentLiftMotorExiSts_write) -> CarCfgForClimaCtrl_CarCfgForVentLiftMotorExiSts/1
  CCMSysServ_Runnable_sys/16(CarCfgForClimaCtrl_CarCfgForWadingAssistSystem_write) -> CarCfgForClimaCtrl_CarCfgForWadingAssistSystem/1
  CCMSysServ_Runnable_sys/17(CarCfgForClimaCtrl_CarCfgWindFollowHumanMov_write) -> CarCfgForClimaCtrl_CarCfgWindFollowHumanMov/1
  CCMSysServ_Runnable_sys/18(HvacCfgForClimaCtrl_HvacCfgForAQSTypes_write) -> HvacCfgForClimaCtrl_HvacCfgForAQSTypes/1
  CCMSysServ_Runnable_sys/19(HvacCfgForClimaCtrl_HvacCfgForHvacLowSideRETXVESts_write) -> HvacCfgForClimaCtrl_HvacCfgForHvacLowSideRETXVESts/1
  CCMSysServ_Runnable_sys/20(HvacCfgForClimaCtrl_HvacCfgForHvacReBlowerType_write) -> HvacCfgForClimaCtrl_HvacCfgForHvacReBlowerType/1
  CCMSysServ_Runnable_sys/21(HvacCfgForClimaCtrl_HvacCfgForHvacReVentPstn_write) -> HvacCfgForClimaCtrl_HvacCfgForHvacReVentPstn/1
  CCMSysServ_Runnable_sys/22(HvacCfgForClimaCtrl_HvacCfgForHvacSnsrRCmptmtESts_write) -> HvacCfgForClimaCtrl_HvacCfgForHvacSnsrRCmptmtESts/1
  CCMSysServ_Runnable_sys/23(HvacCfgForClimaCtrl_HvacCfgForHvacSnsrReHexESts_write) -> HvacCfgForClimaCtrl_HvacCfgForHvacSnsrReHexESts/1
  CCMSysServ_Runnable_sys/24(HvacCfgReqVersionReplace_HvacCfgReqVersionReplace_write) -> HvacCfgReqVersionReplace_HvacCfgReqVersionReplace/1
  CCMSysServ_Runnable_sys/25(SysServCarCfgSts_SysServCCPLin2AQSSts_write) -> SysServCarCfgSts_SysServCCPLin2AQSSts/1
  CCMSysServ_Runnable_sys/26(SysServCarCfgSts_SysServDUBlowerPwmSignTypeSts_write) -> SysServCarCfgSts_SysServDUBlowerPwmSignTypeSts/1
  CCMSysServ_Runnable_sys/27(SysServCarCfgSts_SysServDULin4HFAFStsH_write) -> SysServCarCfgSts_SysServDULin4HFAFStsH/1
  CCMSysServ_Runnable_sys/28(SysServCarCfgSts_SysServDUReBlowerPwmSignSts_write) -> SysServCarCfgSts_SysServDUReBlowerPwmSignSts/1
  CCMSysServ_Runnable_sys/29(SysServCarCfgSts_SysServDUReBlowerPwmSts_write) -> SysServCarCfgSts_SysServDUReBlowerPwmSts/1
  CCMSysServ_Runnable_sys/30(SysServCarCfgSts_SysServFloatingMotorSts_write) -> SysServCarCfgSts_SysServFloatingMotorSts/1
  CCMSysServ_Runnable_sys/31(SysServCarCfgSts_SysServFrntHEXSnsrPosn_write) -> SysServCarCfgSts_SysServFrntHEXSnsrPosn/1
  CCMSysServ_Runnable_sys/32(SysServCarCfgSts_SysServLowSideRETXVSts_write) -> SysServCarCfgSts_SysServLowSideRETXVSts/1
  CCMSysServ_Runnable_sys/33(SysServCarCfgSts_SysServReHEXSnsrPosn_write) -> SysServCarCfgSts_SysServReHEXSnsrPosn/1
  CCMSysServ_Runnable_sys/34(SysServCarCfgSts_SysServRHFASts_write) -> SysServCarCfgSts_SysServRHFASts/1
  CCMSysServ_Runnable_sys/35(SysServCarCfgSts_SysServSnsrForFCmptmtTType_write) -> SysServCarCfgSts_SysServSnsrForFCmptmtTType/1
  CCMSysServ_Runnable_sys/36(CarCfgForClimaCtrl_CarCfgForAutDemst_write) -> CarCfgForClimaCtrl_CarCfgForAutDemst/1
  CCMSysServ_Runnable_sys/37(CarCfgForClimaCtrl_CarCfgForAirClng_write) -> CarCfgForClimaCtrl_CarCfgForAirClng/1
  CCMSysServ_Runnable_sys/38(CarCfgForClimaCtrl_CarCfgForAirClnrAdv_write) -> CarCfgForClimaCtrl_CarCfgForAirClnrAdv/1
  CCMSysServ_Runnable_sys/39(CarCfgForClimaCtrl_CarCfgForAirFrag_write) -> CarCfgForClimaCtrl_CarCfgForAirFrag/1
  CCMSysServ_Runnable_sys/40(CarCfgForClimaCtrl_CarCfgForAirMtrlSnsr_write) -> CarCfgForClimaCtrl_CarCfgForAirMtrlSnsr/1
  CCMSysServ_Runnable_sys/41(CarCfgForClimaCtrl_CarCfgForAirQly_write) -> CarCfgForClimaCtrl_CarCfgForAirQly/1
  CCMSysServ_Runnable_sys/42(CarCfgForClimaCtrl_CarCfgForAmntOfSeat_write) -> CarCfgForClimaCtrl_CarCfgForAmntOfSeat/1
  CCMSysServ_Runnable_sys/43(CarCfgForClimaCtrl_CarCfgForAuxWtrPmp_write) -> CarCfgForClimaCtrl_CarCfgForAuxWtrPmp/1
  CCMSysServ_Runnable_sys/44(CarCfgForClimaCtrl_CarCfgForBypVlvInHvac_write) -> CarCfgForClimaCtrl_CarCfgForBypVlvInHvac/1
  CCMSysServ_Runnable_sys/45(CarCfgForClimaCtrl_CarCfgForClsVlvInHvac_write) -> CarCfgForClimaCtrl_CarCfgForClsVlvInHvac/1
  CCMSysServ_Runnable_sys/46(CarCfgForClimaCtrl_CarCfgForCmprAcTyp_write) -> CarCfgForClimaCtrl_CarCfgForCmprAcTyp/1
  CCMSysServ_Runnable_sys/47(CarCfgForClimaCtrl_CarCfgForCmptmtClng_write) -> CarCfgForClimaCtrl_CarCfgForCmptmtClng/1
  CCMSysServ_Runnable_sys/48(CarCfgForClimaCtrl_CarCfgForElecVentAdjmt_write) -> CarCfgForClimaCtrl_CarCfgForElecVentAdjmt/1
  CCMSysServ_Runnable_sys/49(CarCfgForClimaCtrl_CarCfgForFlcTyp_write) -> CarCfgForClimaCtrl_CarCfgForFlcTyp/1
  CCMSysServ_Runnable_sys/50(CarCfgForClimaCtrl_CarCfgForFoldCSD_write) -> CarCfgForClimaCtrl_CarCfgForFoldCSD/1
  CCMSysServ_Runnable_sys/51(CarCfgForClimaCtrl_CarCfgForFuTyp_write) -> CarCfgForClimaCtrl_CarCfgForFuTyp/1
  CCMSysServ_Runnable_sys/52(CarCfgForClimaCtrl_CarCfgForGlassTyp_write) -> CarCfgForClimaCtrl_CarCfgForGlassTyp/1
  CCMSysServ_Runnable_sys/53(CarCfgForClimaCtrl_CarCfgForHeadRadar_write) -> CarCfgForClimaCtrl_CarCfgForHeadRadar/1
  CCMSysServ_Runnable_sys/54(CarCfgForClimaCtrl_CarCfgForHeatPmp_write) -> CarCfgForClimaCtrl_CarCfgForHeatPmp/1
  CCMSysServ_Runnable_sys/55(CarCfgForClimaCtrl_CarCfgForHeatrDurgDrvg_write) -> CarCfgForClimaCtrl_CarCfgForHeatrDurgDrvg/1
  CCMSysServ_Runnable_sys/56(CarCfgForClimaCtrl_CarCfgForHeatrTyp_write) -> CarCfgForClimaCtrl_CarCfgForHeatrTyp/1
  CCMSysServ_Runnable_sys/57(CarCfgForClimaCtrl_CarCfgForHvacFilTyp_write) -> CarCfgForClimaCtrl_CarCfgForHvacFilTyp/1
  CCMSysServ_Runnable_sys/58(CarCfgForClimaCtrl_CarCfgForHvacFlrSnsrForT_write) -> CarCfgForClimaCtrl_CarCfgForHvacFlrSnsrForT/1
  CCMSysServ_Runnable_sys/59(CarCfgForClimaCtrl_CarCfgForHvacHeatrSnsrT_write) -> CarCfgForClimaCtrl_CarCfgForHvacHeatrSnsrT/1
  CCMSysServ_Runnable_sys/60(CarCfgForClimaCtrl_CarCfgForHvacRe_write) -> CarCfgForClimaCtrl_CarCfgForHvacRe/1
  CCMSysServ_Runnable_sys/61(CarCfgForClimaCtrl_CarCfgForHvacTyp_write) -> CarCfgForClimaCtrl_CarCfgForHvacTyp/1
  CCMSysServ_Runnable_sys/62(CarCfgForClimaCtrl_CarCfgForLoVoltElecCfg_write) -> CarCfgForClimaCtrl_CarCfgForLoVoltElecCfg/1
  CCMSysServ_Runnable_sys/63(CarCfgForClimaCtrl_CarCfgForNavSysandIntnmap_write) -> CarCfgForClimaCtrl_CarCfgForNavSysandIntnmap/1
  CCMSysServ_Runnable_sys/64(CarCfgForClimaCtrl_CarCfgForNrOfDoor_write) -> CarCfgForClimaCtrl_CarCfgForNrOfDoor/1
  CCMSysServ_Runnable_sys/65(CarCfgForClimaCtrl_CarCfgForPipeInFuTank_write) -> CarCfgForClimaCtrl_CarCfgForPipeInFuTank/1
  CCMSysServ_Runnable_sys/66(CarCfgForClimaCtrl_CarCfgForPreClng_write) -> CarCfgForClimaCtrl_CarCfgForPreClng/1
  CCMSysServ_Runnable_sys/67(CarCfgForClimaCtrl_CarCfgForPrkgCDSNotAvl_write) -> CarCfgForClimaCtrl_CarCfgForPrkgCDSNotAvl/1
  CCMSysServ_Runnable_sys/68(CarCfgForClimaCtrl_CarCfgForPrkgClima_write) -> CarCfgForClimaCtrl_CarCfgForPrkgClima/1
  CCMSysServ_Runnable_sys/69(CarCfgForClimaCtrl_CarCfgForPrpsnTyp_write) -> CarCfgForClimaCtrl_CarCfgForPrpsnTyp/1
  CCMSysServ_Runnable_sys/70(CarCfgForClimaCtrl_CarCfgForRemEngStrtPreCdn_write) -> CarCfgForClimaCtrl_CarCfgForRemEngStrtPreCdn/1
  CCMSysServ_Runnable_sys/71(CarCfgForClimaCtrl_CarCfgForRoofHatch_write) -> CarCfgForClimaCtrl_CarCfgForRoofHatch/1
  CCMSysServ_Runnable_sys/72(CarCfgForClimaCtrl_CarCfgForSeatHeatgCal_write) -> CarCfgForClimaCtrl_CarCfgForSeatHeatgCal/1
  CCMSysServ_Runnable_sys/73(CarCfgForClimaCtrl_CarCfgForSeatHeatgForRowFirst_write) -> CarCfgForClimaCtrl_CarCfgForSeatHeatgForRowFirst/1
  CCMSysServ_Runnable_sys/74(CarCfgForClimaCtrl_CarCfgForSeatHeatgForRowSec_write) -> CarCfgForClimaCtrl_CarCfgForSeatHeatgForRowSec/1
  CCMSysServ_Runnable_sys/75(CarCfgForClimaCtrl_CarCfgForSeatVentnForRowFirst_write) -> CarCfgForClimaCtrl_CarCfgForSeatVentnForRowFirst/1
  CCMSysServ_Runnable_sys/76(CarCfgForClimaCtrl_CarCfgForSeatVentnForRowSec_write) -> CarCfgForClimaCtrl_CarCfgForSeatVentnForRowSec/1
  CCMSysServ_Runnable_sys/77(CarCfgForClimaCtrl_CarCfgForSecRowVentnCenter_write) -> CarCfgForClimaCtrl_CarCfgForSecRowVentnCenter/1
  CCMSysServ_Runnable_sys/78(CarCfgForClimaCtrl_CarCfgForSecRowVentnSide_write) -> CarCfgForClimaCtrl_CarCfgForSecRowVentnSide/1
  CCMSysServ_Runnable_sys/79(CarCfgForClimaCtrl_CarCfgForSmallFlowCyc_write) -> CarCfgForClimaCtrl_CarCfgForSmallFlowCyc/1
  CCMSysServ_Runnable_sys/80(CarCfgForClimaCtrl_CarCfgForSmkSnsr_write) -> CarCfgForClimaCtrl_CarCfgForSmkSnsr/1
  CCMSysServ_Runnable_sys/81(CarCfgForClimaCtrl_CarCfgForSnsrForCmptmtT_write) -> CarCfgForClimaCtrl_CarCfgForSnsrForCmptmtT/1
  CCMSysServ_Runnable_sys/82(CarCfgForClimaCtrl_CarCfgForSnsrHumPrsnt_write) -> CarCfgForClimaCtrl_CarCfgForSnsrHumPrsnt/1
  CCMSysServ_Runnable_sys/83(CarCfgForClimaCtrl_CarCfgForStartDevice_write) -> CarCfgForClimaCtrl_CarCfgForStartDevice/1
  CCMSysServ_Runnable_sys/84(CarCfgForClimaCtrl_CarCfgForSteerWhlHeatg_write) -> CarCfgForClimaCtrl_CarCfgForSteerWhlHeatg/1
  CCMSysServ_Runnable_sys/85(CarCfgForClimaCtrl_CarCfgForSteerWhlPosn_write) -> CarCfgForClimaCtrl_CarCfgForSteerWhlPosn/1
  CCMSysServ_Runnable_sys/86(CarCfgForClimaCtrl_CarCfgForSunSnsr_write) -> CarCfgForClimaCtrl_CarCfgForSunSnsr/1
  CCMSysServ_Runnable_sys/87(CarCfgForClimaCtrl_CarCfgForTelmNodTyp_write) -> CarCfgForClimaCtrl_CarCfgForTelmNodTyp/1
  CCMSysServ_Runnable_sys/88(CarCfgForClimaCtrl_CarCfgForThermMngt_write) -> CarCfgForClimaCtrl_CarCfgForThermMngt/1
  CCMSysServ_Runnable_sys/89(CarCfgForClimaCtrl_CarCfgForVehTyp_write) -> CarCfgForClimaCtrl_CarCfgForVehTyp/1
  CCMSysServ_Runnable_sys/90(CarCfgForClimaCtrl_CarCfgForVgaMotorExistSts_write) -> CarCfgForClimaCtrl_CarCfgForVgaMotorExistSts/1
  CCMSysServ_Runnable_sys/91(CarCfgForClimaCtrl_CarCfgForWindHeatd_write) -> CarCfgForClimaCtrl_CarCfgForWindHeatd/1
  CCMSysServ_Runnable_sys/92(HvacCfgForClimaCtrl_HvacCfgForHvacFrontBlowerType_write) -> HvacCfgForClimaCtrl_HvacCfgForHvacFrontBlowerType/1
  CCMSysServ_Runnable_sys/93(HvacCfgForClimaCtrl_HvacCfgForHvacMiscExistSts_write) -> HvacCfgForClimaCtrl_HvacCfgForHvacMiscExistSts/1
  CCMSysServ_Runnable_sys/94(HvacCfgForClimaCtrl_HvacCfgForHvacMotorExistSts_write) -> HvacCfgForClimaCtrl_HvacCfgForHvacMotorExistSts/1
  CCMSysServ_Runnable_sys/95(HvacCfgForClimaCtrl_HvacCfgForHvacSensorExistSts_write) -> HvacCfgForClimaCtrl_HvacCfgForHvacSensorExistSts/1
  CCMSysServ_Runnable_sys/96(SysServCarCfgSts_SysServCarID_write) -> SysServCarCfgSts_SysServCarID/1
  CCMSysServ_Runnable_sys/97(SysServCarCfgSts_SysServCCPAQSSts_write) -> SysServCarCfgSts_SysServCCPAQSSts/1
  CCMSysServ_Runnable_sys/98(SysServCarCfgSts_SysServCCPBGMNum_write) -> SysServCarCfgSts_SysServCCPBGMNum/1
  CCMSysServ_Runnable_sys/99(SysServCarCfgSts_SysServCCPHEXSensorSts_write) -> SysServCarCfgSts_SysServCCPHEXSensorSts/1
  CCMSysServ_Runnable_sys/100(SysServCarCfgSts_SysServCCPLin2AFUSts_write) -> SysServCarCfgSts_SysServCCPLin2AFUSts/1
  CCMSysServ_Runnable_sys/101(SysServCarCfgSts_SysServCCPLin2AIUSts_write) -> SysServCarCfgSts_SysServCCPLin2AIUSts/1
  CCMSysServ_Runnable_sys/102(SysServCarCfgSts_SysServCCPLin2CDSSts_write) -> SysServCarCfgSts_SysServCCPLin2CDSSts/1
  CCMSysServ_Runnable_sys/103(SysServCarCfgSts_SysServCCPLin2PMSISts_write) -> SysServCarCfgSts_SysServCCPLin2PMSISts/1
  CCMSysServ_Runnable_sys/104(SysServCarCfgSts_SysServCCPLin3VGASts_write) -> SysServCarCfgSts_SysServCCPLin3VGASts/1
  CCMSysServ_Runnable_sys/105(SysServCarCfgSts_SysServDUAirVentSnrSts_write) -> SysServCarCfgSts_SysServDUAirVentSnrSts/1
  CCMSysServ_Runnable_sys/106(SysServCarCfgSts_SysServDUBlowerPwmSignSts_write) -> SysServCarCfgSts_SysServDUBlowerPwmSignSts/1
  CCMSysServ_Runnable_sys/107(SysServCarCfgSts_SysServDUBlowerPwmSts_write) -> SysServCarCfgSts_SysServDUBlowerPwmSts/1
  CCMSysServ_Runnable_sys/108(SysServCarCfgSts_SysServDUDCDefrostSts_write) -> SysServCarCfgSts_SysServDUDCDefrostSts/1
  CCMSysServ_Runnable_sys/109(SysServCarCfgSts_SysServDUDCFLMdeSts_write) -> SysServCarCfgSts_SysServDUDCFLMdeSts/1
  CCMSysServ_Runnable_sys/110(SysServCarCfgSts_SysServDUDCModeSts_write) -> SysServCarCfgSts_SysServDUDCModeSts/1
  CCMSysServ_Runnable_sys/111(SysServCarCfgSts_SysServDUDCRecSts_write) -> SysServCarCfgSts_SysServDUDCRecSts/1
  CCMSysServ_Runnable_sys/112(SysServCarCfgSts_SysServDUDCSModeSts_write) -> SysServCarCfgSts_SysServDUDCSModeSts/1
  CCMSysServ_Runnable_sys/113(SysServCarCfgSts_SysServDUDCSTLSts_write) -> SysServCarCfgSts_SysServDUDCSTLSts/1
  CCMSysServ_Runnable_sys/114(SysServCarCfgSts_SysServDUDCTLSts_write) -> SysServCarCfgSts_SysServDUDCTLSts/1
  CCMSysServ_Runnable_sys/115(SysServCarCfgSts_SysServDUDCTRSts_write) -> SysServCarCfgSts_SysServDUDCTRSts/1
  CCMSysServ_Runnable_sys/116(SysServCarCfgSts_SysServDUFLFootSts_write) -> SysServCarCfgSts_SysServDUFLFootSts/1
  CCMSysServ_Runnable_sys/117(SysServCarCfgSts_SysServDUFLVentSts_write) -> SysServCarCfgSts_SysServDUFLVentSts/1
  CCMSysServ_Runnable_sys/118(SysServCarCfgSts_SysServDUFRFootSts_write) -> SysServCarCfgSts_SysServDUFRFootSts/1
  CCMSysServ_Runnable_sys/119(SysServCarCfgSts_SysServDUFRVentSts_write) -> SysServCarCfgSts_SysServDUFRVentSts/1
  CCMSysServ_Runnable_sys/120(SysServCarCfgSts_SysServDUIncarMotorSts_write) -> SysServCarCfgSts_SysServDUIncarMotorSts/1
  CCMSysServ_Runnable_sys/121(SysServCarCfgSts_SysServDULin1HBMFSts_write) -> SysServCarCfgSts_SysServDULin1HBMFSts/1
  CCMSysServ_Runnable_sys/122(SysServCarCfgSts_SysServDULin4HFAFSts_write) -> SysServCarCfgSts_SysServDULin4HFAFSts/1
  CCMSysServ_Runnable_sys/123(SysServCarCfgSts_SysServDUSFLFootSts_write) -> SysServCarCfgSts_SysServDUSFLFootSts/1
  CCMSysServ_Runnable_sys/124(SysServCarCfgSts_SysServDUSFLVentSts_write) -> SysServCarCfgSts_SysServDUSFLVentSts/1
  CCMSysServ_Runnable_sys/125(SysServCarCfgSts_SysServDUSFRFootSts_write) -> SysServCarCfgSts_SysServDUSFRFootSts/1
  CCMSysServ_Runnable_sys/126(SysServCarCfgSts_SysServDUSFRVentSts_write) -> SysServCarCfgSts_SysServDUSFRVentSts/1
  CCMSysServ_Runnable_sys/127(SysServCarCfgSts_SysServDUSnsrForFCmptmtTSts_write) -> SysServCarCfgSts_SysServDUSnsrForFCmptmtTSts/1
  CCMSysServ_Runnable_sys/128(SysServCarCfgSts_SysServDUSnsrForRair_write) -> SysServCarCfgSts_SysServDUSnsrForRair/1
  CCMSysServ_Runnable_sys/129(SysServCarCfgSts_SysServDUSnsrForRCmptmtTSts_write) -> SysServCarCfgSts_SysServDUSnsrForRCmptmtTSts/1
  CCMSysServ_Runnable_sys/130(SysServEcuSlpDelayFinish_EcuSlpDelayFinishFlg_write) -> SysServEcuSlpDelayFinish_EcuSlpDelayFinishFlg/1
  BswLifeCycle_LifeCycle/1 -> CCMSysServ_Runnable_sys/625(BswLifeCycle_LifeCycle_read)
  CCMSysServ_Runnable_sys/131(dp_LifeCycle_LifeCycle_write) -> dp_LifeCycle_LifeCycle/1
  Vin_Vin/1 -> [Vin_Vin_Tag]
  [Vin_Vin_Tag] -> CCMSysServ_Runnable_sys/624(Vin_Vin_read)
  [Vin_Vin_Tag] -> CCMSysServ_Vin_sys/1(Vin_Vin_read)
  VehModMngtGlbSafe1_VehModMngtGlbSafe1/1 -> [VehModMngtGlbSafe1_VehModMngtGlbSafe1_Tag]
  [VehModMngtGlbSafe1_VehModMngtGlbSafe1_Tag] -> CCMSysServ_Runnable_sys/623(VehModMngtGlbSafe1_VehModMngtGlbSafe1_read)
  [VehModMngtGlbSafe1_VehModMngtGlbSafe1_Tag] -> CCMSysServ_VehModMngtGlbSafe1_sys/1(VehModMngtGlbSafe1_VehModMngtGlbSafe1_read)
  VehCfgPrmExt_VehCfgPrmExt/1 -> [VehCfgPrmExt_VehCfgPrmExt_Tag]
  [VehCfgPrmExt_VehCfgPrmExt_Tag] -> CCMSysServ_Runnable_sys/622(VehCfgPrmExt_VehCfgPrmExt_read)
  [VehCfgPrmExt_VehCfgPrmExt_Tag] -> CCMSysServ_VehCfgPrmExt_sys/1(VehCfgPrmExt_VehCfgPrmExt_read)
  VehCfgPrm_VehCfgPrm/1 -> [VehCfgPrm_VehCfgPrm_Tag]
  [VehCfgPrm_VehCfgPrm_Tag] -> CCMSysServ_Runnable_sys/621(VehCfgPrm_VehCfgPrm_read)
  [VehCfgPrm_VehCfgPrm_Tag] -> CCMSysServ_VehCfgPrm_sys/1(VehCfgPrm_VehCfgPrm_read)
  CCMSysServ_Runnable/1 -> CCMSysServ_Runnable_sys/Trigger
  CCMSysServ_VehCfgPrm/1 -> CCMSysServ_VehCfgPrm_sys/Trigger
  CCMSysServ_VehCfgPrmExt/1 -> CCMSysServ_VehCfgPrmExt_sys/Trigger
  CCMSysServ_VehModMngtGlbSafe1/1 -> CCMSysServ_VehModMngtGlbSafe1_sys/Trigger
  CCMSysServ_Vin/1 -> CCMSysServ_Vin_sys/Trigger
